simulink model "ClimaManModMgr"  (R2022b) (continued)
== system ClimaManModMgr == (continued)
  ClimaAutCtrldFlowPredForRowSecAtFlrAtStaby_ClimaAutCtrldFlowPredForRowSecAtFlrAtStaby/1 -> ClimaManModMgr_Runnable_sys/14(ClimaAutCtrldFlowPredForRowSecAtFlrAtStaby_ClimaAutCtrldFlowPredForRowSecAtFlrAtStaby_read)
  ClimaAutCtrldFlowPredForRowSecAtFlrNoLim_ClimaAutCtrldFlowPredForRowSecAtFlrNoLim/1 -> ClimaManModMgr_Runnable_sys/15(ClimaAutCtrldFlowPredForRowSecAtFlrNoLim_ClimaAutCtrldFlowPredForRowSecAtFlrNoLim_read)
  ClimaAutCtrldFlowPredForRowSecAtVentAtStaby_ClimaAutCtrldFlowPredForRowSecAtVentAtStaby/1 -> ClimaManModMgr_Runnable_sys/16(ClimaAutCtrldFlowPredForRowSecAtVentAtStaby_ClimaAutCtrldFlowPredForRowSecAtVentAtStaby_read)
  ClimaAutCtrldFlowPredForRowSecAtVentNoLim_ClimaAutCtrldFlowPredForRowSecAtVentNoLim/1 -> ClimaManModMgr_Runnable_sys/17(ClimaAutCtrldFlowPredForRowSecAtVentNoLim_ClimaAutCtrldFlowPredForRowSecAtVentNoLim_read)
  ClimaAutCtrldFlowReqForDefrst_ClimaAutCtrldFlowReqForDefrst/1 -> ClimaManModMgr_Runnable_sys/18(ClimaAutCtrldFlowReqForDefrst_ClimaAutCtrldFlowReqForDefrst_read)
  ClimaAutCtrldFlowReqForDefrst_ClimaAutCtrldFlowReqForDefrstLe/1 -> ClimaManModMgr_Runnable_sys/19(ClimaAutCtrldFlowReqForDefrst_ClimaAutCtrldFlowReqForDefrstLe_read)
  ClimaAutCtrldFlowReqForDefrst_ClimaAutCtrldFlowReqForDefrstRi/1 -> ClimaManModMgr_Runnable_sys/20(ClimaAutCtrldFlowReqForDefrst_ClimaAutCtrldFlowReqForDefrstRi_read)
  ClimaAutCtrldFlowReqForRowFirstAtFlr_ClimaAutCtrldFlowReqForRowFirstAtFlr/1 -> ClimaManModMgr_Runnable_sys/21(ClimaAutCtrldFlowReqForRowFirstAtFlr_ClimaAutCtrldFlowReqForRowFirstAtFlr_read)
  ClimaAutCtrldFlowReqForRowFirstAtFlr_ClimaAutCtrldFlowReqForRowFirstAtFlrRi/1 -> ClimaManModMgr_Runnable_sys/22(ClimaAutCtrldFlowReqForRowFirstAtFlr_ClimaAutCtrldFlowReqForRowFirstAtFlrRi_read)
  ClimaAutCtrldFlowReqForRowSecAtFlr_ClimaAutCtrldFlowReqForRowSecAtFlr/1 -> ClimaManModMgr_Runnable_sys/23(ClimaAutCtrldFlowReqForRowSecAtFlr_ClimaAutCtrldFlowReqForRowSecAtFlr_read)
  ClimaAutCtrldFlowReqForRowSecAtFlr_ClimaAutCtrldFlowReqForRowSecAtFlrLe/1 -> ClimaManModMgr_Runnable_sys/24(ClimaAutCtrldFlowReqForRowSecAtFlr_ClimaAutCtrldFlowReqForRowSecAtFlrLe_read)
  ClimaAutCtrldFlowReqForRowSecAtFlr_ClimaAutCtrldFlowReqForRowSecAtFlrRi/1 -> ClimaManModMgr_Runnable_sys/25(ClimaAutCtrldFlowReqForRowSecAtFlr_ClimaAutCtrldFlowReqForRowSecAtFlrRi_read)
  ClimaAutCtrldFlowReqForRowSecAtVent_ClimaAutCtrldFlowReqForRowSecAtVent/1 -> ClimaManModMgr_Runnable_sys/26(ClimaAutCtrldFlowReqForRowSecAtVent_ClimaAutCtrldFlowReqForRowSecAtVent_read)
  ClimaAutCtrldFlowReqForRowSecAtVent_ClimaAutCtrldFlowReqForRowSecAtVentLe/1 -> ClimaManModMgr_Runnable_sys/27(ClimaAutCtrldFlowReqForRowSecAtVent_ClimaAutCtrldFlowReqForRowSecAtVentLe_read)
  ClimaAutCtrldFlowReqForRowSecAtVent_ClimaAutCtrldFlowReqForRowSecAtVentRi/1 -> ClimaManModMgr_Runnable_sys/28(ClimaAutCtrldFlowReqForRowSecAtVent_ClimaAutCtrldFlowReqForRowSecAtVentRi_read)
  ClimaCfgForCarTyp1_ClimaCfgForCarTyp1/1 -> ClimaManModMgr_Runnable_sys/29(ClimaCfgForCarTyp1_ClimaCfgForCarTyp1_read)
  ClimaCfgForClimaCtrlMan1_ClimaCfgForClimaCtrlMan1/1 -> ClimaManModMgr_Runnable_sys/30(ClimaCfgForClimaCtrlMan1_ClimaCfgForClimaCtrlMan1_read)
  ClimaCfgForExtVentnClimaCtrl_ClimaCfgForExtVentnClimaCtrl/1 -> ClimaManModMgr_Runnable_sys/31(ClimaCfgForExtVentnClimaCtrl_ClimaCfgForExtVentnClimaCtrl_read)
  ClimaCfgForFanPotiCtrld1_ClimaCfgForFanPotiCtrld1/1 -> ClimaManModMgr_Runnable_sys/32(ClimaCfgForFanPotiCtrld1_ClimaCfgForFanPotiCtrld1_read)
  ClimaCfgForHeatrTyp1_ClimaCfgForHeatrTyp1/1 -> ClimaManModMgr_Runnable_sys/33(ClimaCfgForHeatrTyp1_ClimaCfgForHeatrTyp1_read)
  ClimaCfgForNrOfFanLvlSetg1_ClimaCfgForNrOfFanLvlSetg1/1 -> ClimaManModMgr_Runnable_sys/34(ClimaCfgForNrOfFanLvlSetg1_ClimaCfgForNrOfFanLvlSetg1_read)
  ClimaCfgForNrOfTSetg1_ClimaCfgForNrOfTSetg1/1 -> ClimaManModMgr_Runnable_sys/35(ClimaCfgForNrOfTSetg1_ClimaCfgForNrOfTSetg1_read)
  ClimaCfgForVehElec1_ClimaCfgForVehElec1/1 -> ClimaManModMgr_Runnable_sys/36(ClimaCfgForVehElec1_ClimaCfgForVehElec1_read)
  ClimaCfgForVehHev1_ClimaCfgForVehHev1/1 -> ClimaManModMgr_Runnable_sys/37(ClimaCfgForVehHev1_ClimaCfgForVehHev1_read)
  ClimaCfgForVehHyb1_ClimaCfgForVehHyb1/1 -> ClimaManModMgr_Runnable_sys/38(ClimaCfgForVehHyb1_ClimaCfgForVehHyb1_read)
  ClimaCtrlActvNgcc_ClimaCtrlActv/1 -> ClimaManModMgr_Runnable_sys/39(ClimaCtrlActvNgcc_ClimaCtrlActv_read)
  ClimaCtrlForEngStbActv_ClimaCtrlForEngStbActv/1 -> ClimaManModMgr_Runnable_sys/40(ClimaCtrlForEngStbActv_ClimaCtrlForEngStbActv_read)
  ClimaStStrtUp_ClimaStStrtUp/1 -> ClimaManModMgr_Runnable_sys/41(ClimaStStrtUp_ClimaStStrtUp_read)
  CmptmtAirTEstimdAtRowFirst_CmptmtAirTEstimdAtRowFirst/1 -> ClimaManModMgr_Runnable_sys/42(CmptmtAirTEstimdAtRowFirst_CmptmtAirTEstimdAtRowFirst_read)
  CmptmtAirTEstimdAtRowSec_CmptmtAirTEstimdAtRowSec/1 -> ClimaManModMgr_Runnable_sys/43(CmptmtAirTEstimdAtRowSec_CmptmtAirTEstimdAtRowSec_read)
  CnvincModActv_CnvincModActv/1 -> ClimaManModMgr_Runnable_sys/44(CnvincModActv_CnvincModActv_read)
  CooltTInHeatElm_CooltTInHeatElm/1 -> ClimaManModMgr_Runnable_sys/45(CooltTInHeatElm_CooltTInHeatElm_read)
  DrvrLeElecAirVentnSts_DrvrLeElecAirVentnSts/1 -> ClimaManModMgr_Runnable_sys/46(DrvrLeElecAirVentnSts_DrvrLeElecAirVentnSts_read)
  DrvrRiElecAirVentnSts_DrvrRiElecAirVentnSts/1 -> ClimaManModMgr_Runnable_sys/47(DrvrRiElecAirVentnSts_DrvrRiElecAirVentnSts_read)
  EcoModForClimaActv_EcoModForClimaActv/1 -> ClimaManModMgr_Runnable_sys/48(EcoModForClimaActv_EcoModForClimaActv_read)
  ExtVentFanVolStsFrmClima_ExtVentFanVolStsFrmClima/1 -> ClimaManModMgr_Runnable_sys/49(ExtVentFanVolStsFrmClima_ExtVentFanVolStsFrmClima_read)
  ExtVentStsFrmClima_ExtVentStsFrmClima/1 -> ClimaManModMgr_Runnable_sys/50(ExtVentStsFrmClima_ExtVentStsFrmClima_read)
  FlowRednAtPwrAllwdLo_FlowRednAtPwrAllwdLo/1 -> ClimaManModMgr_Runnable_sys/51(FlowRednAtPwrAllwdLo_FlowRednAtPwrAllwdLo_read)
  FlowRednAtPwrLvlLo_FlowRednAtPwrLvlLo/1 -> ClimaManModMgr_Runnable_sys/52(FlowRednAtPwrLvlLo_FlowRednAtPwrLvlLo_read)
  HmiCmptmtAirDistbnFrntForClima_HmiCmptmtAirDistbnFrntForClima/1 -> ClimaManModMgr_Runnable_sys/53(HmiCmptmtAirDistbnFrntForClima_HmiCmptmtAirDistbnFrntForClima_read)
  HmiCmptmtAirDistbnFrntForClima_HmiCmptmtAirDistbnFrntRiForClima/1 -> ClimaManModMgr_Runnable_sys/54(HmiCmptmtAirDistbnFrntForClima_HmiCmptmtAirDistbnFrntRiForClima_read)
  HmiCmptmtAirDistbnFrntForClima_HmiCmptmtAirDistbnReForClima/1 -> ClimaManModMgr_Runnable_sys/55(HmiCmptmtAirDistbnFrntForClima_HmiCmptmtAirDistbnReForClima_read)
  HmiCmptmtAirDistbnFrntForClima_HmiCmptmtAirDistbnReRiForClima/1 -> ClimaManModMgr_Runnable_sys/56(HmiCmptmtAirDistbnFrntForClima_HmiCmptmtAirDistbnReRiForClima_read)
  HmiDefrstMaxReqForClima_HmiDefrstMaxReqForClima/1 -> ClimaManModMgr_Runnable_sys/57(HmiDefrstMaxReqForClima_HmiDefrstMaxReqForClima_read)
  HmiHvacFanLvlFrntForClima_HmiHvacFanLvlFrntForClima/1 -> ClimaManModMgr_Runnable_sys/58(HmiHvacFanLvlFrntForClima_HmiHvacFanLvlFrntForClima_read)
  HmiHvacFanLvlFrntForClima_HmiHvacFanLvlFrntRiForClima/1 -> ClimaManModMgr_Runnable_sys/59(HmiHvacFanLvlFrntForClima_HmiHvacFanLvlFrntRiForClima_read)
  HmiHvacFanLvlReForClima_HmiHvacFanLvlReForClima/1 -> ClimaManModMgr_Runnable_sys/60(HmiHvacFanLvlReForClima_HmiHvacFanLvlReForClima_read)
  HmiHvacFanLvlReForClima_HmiHvacFanLvlReRiForClima/1 -> ClimaManModMgr_Runnable_sys/61(HmiHvacFanLvlReForClima_HmiHvacFanLvlReRiForClima_read)
  HmiMaxACReqForClima_HmiMaxACReqForClima/1 -> ClimaManModMgr_Runnable_sys/62(HmiMaxACReqForClima_HmiMaxACReqForClima_read)
  HvacAirMFlowReqMaxAvl_HvacAirMFlowReqMaxAvl/1 -> ClimaManModMgr_Runnable_sys/63(HvacAirMFlowReqMaxAvl_HvacAirMFlowReqMaxAvl_read)
  HvacAirTForRowFirstAtFlrLe_HvacAirTForRowFirstAtFlrLe_HvacAirTForRowFirstAtFlrLe/1 -> ClimaManModMgr_Runnable_sys/64(HvacAirTForRowFirstAtFlrLe_HvacAirTForRowFirstAtFlrLe_HvacAirTForRowFirstAtFlrLe_read)
  HvacAirTForRowFirstAtFlrLeRaw_HvacAirTForRowFirstAtFlrLeRaw/1 -> ClimaManModMgr_Runnable_sys/65(HvacAirTForRowFirstAtFlrLeRaw_HvacAirTForRowFirstAtFlrLeRaw_read)
  PassLeElecAirVentnSts_PassLeElecAirVentnSts/1 -> ClimaManModMgr_Runnable_sys/66(PassLeElecAirVentnSts_PassLeElecAirVentnSts_read)
  PassRiElecAirVentnSts_PassRiElecAirVentnSts/1 -> ClimaManModMgr_Runnable_sys/67(PassRiElecAirVentnSts_PassRiElecAirVentnSts_read)
  PreClngSts_PreClngSts/1 -> ClimaManModMgr_Runnable_sys/68(PreClngSts_PreClngSts_read)
  PrkgClimaActv_PrkgClimaActv/1 -> ClimaManModMgr_Runnable_sys/69(PrkgClimaActv_PrkgClimaActv_read)
  ReLeElecAirVentnSts_ReLeElecAirVentnSts/1 -> ClimaManModMgr_Runnable_sys/70(ReLeElecAirVentnSts_ReLeElecAirVentnSts_read)
  ReRiElecAirVentnSts_ReRiElecAirVentnSts/1 -> ClimaManModMgr_Runnable_sys/71(ReRiElecAirVentnSts_ReRiElecAirVentnSts_read)
  TModCtrlAtRowFirst_TModCtrlAtRowFirst/1 -> ClimaManModMgr_Runnable_sys/72(TModCtrlAtRowFirst_TModCtrlAtRowFirst_read)
  TModCtrlAtRowFirst_TModCtrlAtRowFirstLe/1 -> ClimaManModMgr_Runnable_sys/73(TModCtrlAtRowFirst_TModCtrlAtRowFirstLe_read)
  TModCtrlAtRowFirst_TModCtrlAtRowFirstRi/1 -> ClimaManModMgr_Runnable_sys/74(TModCtrlAtRowFirst_TModCtrlAtRowFirstRi_read)
  VehModMngtGlbSafe1_Ngcc_VehModMngtGlbSafe1/1 -> ClimaManModMgr_Runnable_sys/75(VehModMngtGlbSafe1_Ngcc_VehModMngtGlbSafe1_read)
  VentAndFlrModelForRowFirstLeForModelDial_VentAndFlrModelForRowFirstLeForModelDial/1 -> ClimaManModMgr_Runnable_sys/76(VentAndFlrModelForRowFirstLeForModelDial_VentAndFlrModelForRowFirstLeForModelDial_read)
  VentAndFlrModelForRowFirstRiForModelDial_VentAndFlrModelForRowFirstRiForModelDial/1 -> ClimaManModMgr_Runnable_sys/77(VentAndFlrModelForRowFirstRiForModelDial_VentAndFlrModelForRowFirstRiForModelDial_read)
  VentAndFlrModelForRowSecLeForModelDial_VentAndFlrModelForRowSecLeForModelDial/1 -> ClimaManModMgr_Runnable_sys/78(VentAndFlrModelForRowSecLeForModelDial_VentAndFlrModelForRowSecLeForModelDial_read)
  VentAndFlrModelForRowSecRiForModelDial_VentAndFlrModelForRowSecRiForModelDial/1 -> ClimaManModMgr_Runnable_sys/79(VentAndFlrModelForRowSecRiForModelDial_VentAndFlrModelForRowSecRiForModelDial_read)
  AirTFromHeatgEstimdForCCM_AirTFromHeatgEstimd/1 -> ClimaManModMgr_Runnable_sys/80(AirTFromHeatgEstimdForCCM_AirTFromHeatgEstimd_read)
  AutDemstOperSts_AutDemstOperSts/1 -> ClimaManModMgr_Runnable_sys/81(AutDemstOperSts_AutDemstOperSts_read)
  CdsnProblty_CdsnProblty/1 -> ClimaManModMgr_Runnable_sys/82(CdsnProblty_CdsnProblty_read)
  ClimaAutCtrldFlowReqForRowFirstAtFlr_ClimaAutCtrldFlowReqForRowFirstAtFlrLe/1 -> ClimaManModMgr_Runnable_sys/83(ClimaAutCtrldFlowReqForRowFirstAtFlr_ClimaAutCtrldFlowReqForRowFirstAtFlrLe_read)
  ClimaAutCtrldFlowReqForRowFirstAtVent_ClimaAutCtrldFlowReqForRowFirstAtVent/1 -> ClimaManModMgr_Runnable_sys/84(ClimaAutCtrldFlowReqForRowFirstAtVent_ClimaAutCtrldFlowReqForRowFirstAtVent_read)
  ClimaAutCtrldFlowReqForRowFirstAtVent_ClimaAutCtrldFlowReqForRowFirstAtVentLe/1 -> ClimaManModMgr_Runnable_sys/85(ClimaAutCtrldFlowReqForRowFirstAtVent_ClimaAutCtrldFlowReqForRowFirstAtVentLe_read)
  ClimaAutCtrldFlowReqForRowFirstAtVent_ClimaAutCtrldFlowReqForRowFirstAtVentLeMid/1 -> ClimaManModMgr_Runnable_sys/86(ClimaAutCtrldFlowReqForRowFirstAtVent_ClimaAutCtrldFlowReqForRowFirstAtVentLeMid_read)
  ClimaAutCtrldFlowReqForRowFirstAtVent_ClimaAutCtrldFlowReqForRowFirstAtVentLeSide/1 -> ClimaManModMgr_Runnable_sys/87(ClimaAutCtrldFlowReqForRowFirstAtVent_ClimaAutCtrldFlowReqForRowFirstAtVentLeSide_read)
  ClimaAutCtrldFlowReqForRowFirstAtVent_ClimaAutCtrldFlowReqForRowFirstAtVentRi/1 -> ClimaManModMgr_Runnable_sys/88(ClimaAutCtrldFlowReqForRowFirstAtVent_ClimaAutCtrldFlowReqForRowFirstAtVentRi_read)
  ClimaAutCtrldFlowReqForRowFirstAtVent_ClimaAutCtrldFlowReqForRowFirstAtVentRiMid/1 -> ClimaManModMgr_Runnable_sys/89(ClimaAutCtrldFlowReqForRowFirstAtVent_ClimaAutCtrldFlowReqForRowFirstAtVentRiMid_read)
  ClimaAutCtrldFlowReqForRowFirstAtVent_ClimaAutCtrldFlowReqForRowFirstAtVentRiSide/1 -> ClimaManModMgr_Runnable_sys/90(ClimaAutCtrldFlowReqForRowFirstAtVent_ClimaAutCtrldFlowReqForRowFirstAtVentRiSide_read)
  ClimaCfgForActrModVrnt1_ClimaCfgForActrInd1/1 -> ClimaManModMgr_Runnable_sys/91(ClimaCfgForActrModVrnt1_ClimaCfgForActrInd1_read)
  ClimaCfgForActrModVrnt1_ClimaCfgForActrModVrnt1/1 -> ClimaManModMgr_Runnable_sys/92(ClimaCfgForActrModVrnt1_ClimaCfgForActrModVrnt1_read)
  ClimaCfgForActrModVrnt1_ClimaCfgForActrModVrntCen/1 -> ClimaManModMgr_Runnable_sys/93(ClimaCfgForActrModVrnt1_ClimaCfgForActrModVrntCen_read)
  ClimaCfgForStatrtDevice_ClimaCfgForStartDevice/1 -> ClimaManModMgr_Runnable_sys/94(ClimaCfgForStatrtDevice_ClimaCfgForStartDevice_read)
  FacOfSecVent_FacOfSecVent/1 -> ClimaManModMgr_Runnable_sys/95(FacOfSecVent_FacOfSecVent_read)
  FacOfVentLeFlow_FacOfVentLeFlow/1 -> ClimaManModMgr_Runnable_sys/96(FacOfVentLeFlow_FacOfVentLeFlow_read)
  FacOfVentRiFlow_FacOfVentRiFlow/1 -> ClimaManModMgr_Runnable_sys/97(FacOfVentRiFlow_FacOfVentRiFlow_read)
  FacOfVentSideLeFlow_FacOfVentSideLeFlow/1 -> ClimaManModMgr_Runnable_sys/98(FacOfVentSideLeFlow_FacOfVentSideLeFlow_read)
  FacOfVentSideRiFlow_FacOfVentSideRiFlow/1 -> ClimaManModMgr_Runnable_sys/99(FacOfVentSideRiFlow_FacOfVentSideRiFlow_read)
  FlowRednAtPwrLvlLo1_FlowRednAtPwrLvlLo1/1 -> ClimaManModMgr_Runnable_sys/100(FlowRednAtPwrLvlLo1_FlowRednAtPwrLvlLo1_read)
  HmiCmptmtTSpForClima_HmiCmptmtTSpForClima/1 -> ClimaManModMgr_Runnable_sys/101(HmiCmptmtTSpForClima_HmiCmptmtTSpForClima_read)
  CmptmtTFrntRaw_CmptmtTFrntRaw/1 -> ClimaManModMgr_Runnable_sys/102(CmptmtTFrntRaw_CmptmtTFrntRaw_read)
  ActrFrntReqForRowFirstLeModDistbn_ActrFrntReqForRowFirstLeModDistbn/1 -> ClimaManModMgr_Runnable_sys/103(ActrFrntReqForRowFirstLeModDistbn_ActrFrntReqForRowFirstLeModDistbn_read)
  ActrFrntReqForRowFirstModDistbn_ActrFrntReqForRowFirstModDistbn/1 -> ClimaManModMgr_Runnable_sys/104(ActrFrntReqForRowFirstModDistbn_ActrFrntReqForRowFirstModDistbn_read)
  ActrPosnActForModDistbnRe_ActrPosnActForModDistbnRe/1 -> ClimaManModMgr_Runnable_sys/105(ActrPosnActForModDistbnRe_ActrPosnActForModDistbnRe_read)
  ActrPosnFrntActForRowFirstModDistbn_ActrPosnFrntActForRowFirstModDistbn/1 -> ClimaManModMgr_Runnable_sys/106(ActrPosnFrntActForRowFirstModDistbn_ActrPosnFrntActForRowFirstModDistbn_read)
  ActrPosnFrntActForRowFirstModDistbnLe_ActrPosnFrntActForRowFirstModDistbnLe/1 -> ClimaManModMgr_Runnable_sys/107(ActrPosnFrntActForRowFirstModDistbnLe_ActrPosnFrntActForRowFirstModDistbnLe_read)
  ActvnTiForClimaCtrlRe_ActvnTiForClimaCtrlRe/1 -> ClimaManModMgr_Runnable_sys/108(ActvnTiForClimaCtrlRe_ActvnTiForClimaCtrlRe_read)
  AirCdnAvl_AirCdnAvl/1 -> ClimaManModMgr_Runnable_sys/109(AirCdnAvl_AirCdnAvl_read)
  AirPtcEnadNgcc_AirPtcEnadNgcc/1 -> ClimaManModMgr_Runnable_sys/110(AirPtcEnadNgcc_AirPtcEnadNgcc_read)
  AirPtcModeReqNgcc_AirPtcModeReqNgcc/1 -> ClimaManModMgr_Runnable_sys/111(AirPtcModeReqNgcc_AirPtcModeReqNgcc_read)
  AirTAftHvacDesFirstLe_AirTAftHvacDesFirstLe/1 -> ClimaManModMgr_Runnable_sys/112(AirTAftHvacDesFirstLe_AirTAftHvacDesFirstLe_read)
  AirTAftHvacDesFirstRi_AirTAftHvacDesFirstRi/1 -> ClimaManModMgr_Runnable_sys/113(AirTAftHvacDesFirstRi_AirTAftHvacDesFirstRi_read)
  AirTAftHvacDesRe_AirTAftHvacDesRe/1 -> ClimaManModMgr_Runnable_sys/114(AirTAftHvacDesRe_AirTAftHvacDesRe_read)
  AirTAftHvacDesSecLe_AirTAftHvacDesSecLe/1 -> ClimaManModMgr_Runnable_sys/115(AirTAftHvacDesSecLe_AirTAftHvacDesSecLe_read)
  AirTFromHeatgEstimdFirstLeForCCM_AirTFromHeatgEstimdFirstLeForCCM/1 -> ClimaManModMgr_Runnable_sys/116(AirTFromHeatgEstimdFirstLeForCCM_AirTFromHeatgEstimdFirstLeForCCM_read)
  AirTFromHeatgEstimdFirstRiForCCM_AirTFromHeatgEstimdFirstRiForCCM/1 -> ClimaManModMgr_Runnable_sys/117(AirTFromHeatgEstimdFirstRiForCCM_AirTFromHeatgEstimdFirstRiForCCM_read)
  AirTFromHeatgEstimdReForCCM_AirTFromHeatgEstimdReForCCM/1 -> ClimaManModMgr_Runnable_sys/118(AirTFromHeatgEstimdReForCCM_AirTFromHeatgEstimdReForCCM_read)
  AirTFromHeatgEstimdSecLeForCCM_AirTFromHeatgEstimdSecLeForCCM/1 -> ClimaManModMgr_Runnable_sys/119(AirTFromHeatgEstimdSecLeForCCM_AirTFromHeatgEstimdSecLeForCCM_read)
  AutDemstWthFanReq_AutDemstWthFanReq/1 -> ClimaManModMgr_Runnable_sys/120(AutDemstWthFanReq_AutDemstWthFanReq_read)
  ClimaCfgForAutDemst_ClimaCfgForAutDemst/1 -> ClimaManModMgr_Runnable_sys/121(ClimaCfgForAutDemst_ClimaCfgForAutDemst_read)
  ClimaCfgForErev1_ClimaCfgForErev1/1 -> ClimaManModMgr_Runnable_sys/122(ClimaCfgForErev1_ClimaCfgForErev1_read)
  ClimaCfgForForHeatPmp_ClimaCfgForForHeatPmp/1 -> ClimaManModMgr_Runnable_sys/123(ClimaCfgForForHeatPmp_ClimaCfgForForHeatPmp_read)
  ClimaCfgForHvacRePrsnt1_ClimaCfgForHvacRePrsnt1/1 -> ClimaManModMgr_Runnable_sys/124(ClimaCfgForHvacRePrsnt1_ClimaCfgForHvacRePrsnt1_read)
  ClimaCfgForPTCInFrontHVAC_ClimaCfgForPTCInFrontHVAC/1 -> ClimaManModMgr_Runnable_sys/125(ClimaCfgForPTCInFrontHVAC_ClimaCfgForPTCInFrontHVAC_read)
  ClimaCfgForSnsrHumPrsnt1_ClimaCfgForSnsrHumPrsnt1/1 -> ClimaManModMgr_Runnable_sys/126(ClimaCfgForSnsrHumPrsnt1_ClimaCfgForSnsrHumPrsnt1_read)
  ClimaCfgHvacFrntForRowSecForActrTLe_ClimaCfgHvacFrntForRowSecForActrTLe/1 -> ClimaManModMgr_Runnable_sys/127(ClimaCfgHvacFrntForRowSecForActrTLe_ClimaCfgHvacFrntForRowSecForActrTLe_read)
  ClimaCfgHvacFrntForRowSecForActrTRi_ClimaCfgHvacFrntForRowSecForActrTRi/1 -> ClimaManModMgr_Runnable_sys/128(ClimaCfgHvacFrntForRowSecForActrTRi_ClimaCfgHvacFrntForRowSecForActrTRi_read)
  ClimaCfgReqVersionReplace_ClimaCfgReqVersionReplace/1 -> ClimaManModMgr_Runnable_sys/129(ClimaCfgReqVersionReplace_ClimaCfgReqVersionReplace_read)
  ClimaStStrtUpRe_ClimaStStrtUpRe/1 -> ClimaManModMgr_Runnable_sys/130(ClimaStStrtUpRe_ClimaStStrtUpRe_read)
  CmptmtAirTEstimdExtd_Ngcc_CmptmtAirTEstimdExtd/1 -> ClimaManModMgr_Runnable_sys/131(CmptmtAirTEstimdExtd_Ngcc_CmptmtAirTEstimdExtd_read)
  CmptmtTRearRaw_CmptmtTRearRaw/1 -> ClimaManModMgr_Runnable_sys/132(CmptmtTRearRaw_CmptmtTRearRaw_read)
  CoupldCtrlErrAtRowFirstAvg_CoupldCtrlErrAtRowFirstAvg/1 -> ClimaManModMgr_Runnable_sys/133(CoupldCtrlErrAtRowFirstAvg_CoupldCtrlErrAtRowFirstAvg_read)
  CoupldCtrlErrAtRowFirstLeNgcc_CoupldCtrlErrAtRowFirstLe/1 -> ClimaManModMgr_Runnable_sys/134(CoupldCtrlErrAtRowFirstLeNgcc_CoupldCtrlErrAtRowFirstLe_read)
  CoupldCtrlErrAtRowFirstRiNgcc_CoupldCtrlErrAtRowFirstRi/1 -> ClimaManModMgr_Runnable_sys/135(CoupldCtrlErrAtRowFirstRiNgcc_CoupldCtrlErrAtRowFirstRi_read)
  CoupldCtrlErrAtRowSecAvg_CoupldCtrlErrAtRowSecAvg/1 -> ClimaManModMgr_Runnable_sys/136(CoupldCtrlErrAtRowSecAvg_CoupldCtrlErrAtRowSecAvg_read)
  CoupldCtrlErrAtRowSecLeNgcc_CoupldCtrlErrAtRowSecLe/1 -> ClimaManModMgr_Runnable_sys/137(CoupldCtrlErrAtRowSecLeNgcc_CoupldCtrlErrAtRowSecLe_read)
  CoupldCtrlErrAtRowSecRiNgcc_CoupldCtrlErrAtRowSecRi/1 -> ClimaManModMgr_Runnable_sys/138(CoupldCtrlErrAtRowSecRiNgcc_CoupldCtrlErrAtRowSecRi_read)
  DCDCPwrAllwdForHvacFanRe_DCDCPwrAllwdForHvacFanRe/1 -> ClimaManModMgr_Runnable_sys/139(DCDCPwrAllwdForHvacFanRe_DCDCPwrAllwdForHvacFanRe_read)
  DropTopPosnStsNgcc_DropTopPosnStsNgcc/1 -> ClimaManModMgr_Runnable_sys/140(DropTopPosnStsNgcc_DropTopPosnStsNgcc_read)
  EvaprTFrntFildForClima_EvaprTFrntFildForClima/1 -> ClimaManModMgr_Runnable_sys/141(EvaprTFrntFildForClima_EvaprTFrntFildForClima_read)
  EvaprTReFildForClima_EvaprTReFildForClima/1 -> ClimaManModMgr_Runnable_sys/142(EvaprTReFildForClima_EvaprTReFildForClima_read)
  ExtVentToastFrmClima_Prkg_ExtVentToastFrmClima/1 -> ClimaManModMgr_Runnable_sys/143(ExtVentToastFrmClima_Prkg_ExtVentToastFrmClima_read)
  FlowRednForEngStby_FlowRednForEngStby/1 -> ClimaManModMgr_Runnable_sys/144(FlowRednForEngStby_FlowRednForEngStby_read)
  FogFlashPrevnActv_FogFlashPrevnActv/1 -> ClimaManModMgr_Runnable_sys/145(FogFlashPrevnActv_FogFlashPrevnActv_read)
  FrntHvacBlowerSts_FrntHvacBlowerSts/1 -> ClimaManModMgr_Runnable_sys/146(FrntHvacBlowerSts_FrntHvacBlowerSts_read)
  HmiClimaFrntAutReq_HmiClimaFrntAutReq/1 -> ClimaManModMgr_Runnable_sys/147(HmiClimaFrntAutReq_HmiClimaFrntAutReq_read)
  HvacAirMFlowEstimd_Ngcc_HvacAirMFlowEstimd/1 -> ClimaManModMgr_Runnable_sys/148(HvacAirMFlowEstimd_Ngcc_HvacAirMFlowEstimd_read)
  HvacAirMFlowEstimdForCtrlr_HvacAirMFlowEstimdForCtrlr/1 -> ClimaManModMgr_Runnable_sys/149(HvacAirMFlowEstimdForCtrlr_HvacAirMFlowEstimdForCtrlr_read)
  HvacAirMFlowEstimdForCtrlrRe_HvacAirMFlowEstimdForCtrlrRe/1 -> ClimaManModMgr_Runnable_sys/150(HvacAirMFlowEstimdForCtrlrRe_HvacAirMFlowEstimdForCtrlrRe_read)
  HvacAirMFlowMaxEstimd_HvacAirMFlowMaxEstimd/1 -> ClimaManModMgr_Runnable_sys/151(HvacAirMFlowMaxEstimd_HvacAirMFlowMaxEstimd_read)
  HvacAirMFlowMaxEstimdRe_HvacAirMFlowMaxEstimdRe/1 -> ClimaManModMgr_Runnable_sys/152(HvacAirMFlowMaxEstimdRe_HvacAirMFlowMaxEstimdRe_read)
  HvacAirMFlowReEstimd_Ngcc_HvacAirMFlowReEstimd/1 -> ClimaManModMgr_Runnable_sys/153(HvacAirMFlowReEstimd_Ngcc_HvacAirMFlowReEstimd_read)
  HvacAirTEstimdAtRowFirstLe_HvacAirTEstimdAtRowFirstLe/1 -> ClimaManModMgr_Runnable_sys/154(HvacAirTEstimdAtRowFirstLe_HvacAirTEstimdAtRowFirstLe_read)
  HvacAirTEstimdAtRowFirstRi_HvacAirTEstimdAtRowFirstRi/1 -> ClimaManModMgr_Runnable_sys/155(HvacAirTEstimdAtRowFirstRi_HvacAirTEstimdAtRowFirstRi_read)
  HvacAirTEstimdAtRowSecLe_HvacAirTEstimdAtRowSecLe/1 -> ClimaManModMgr_Runnable_sys/156(HvacAirTEstimdAtRowSecLe_HvacAirTEstimdAtRowSecLe_read)
  HvacAirTEstimdAtRowSecRi_HvacAirTEstimdAtRowSecRi/1 -> ClimaManModMgr_Runnable_sys/157(HvacAirTEstimdAtRowSecRi_HvacAirTEstimdAtRowSecRi_read)
  HvacAirTFildForRowFirstAtDefrstLe_HvacAirTFildForRowFirstAtDefrstLe/1 -> ClimaManModMgr_Runnable_sys/158(HvacAirTFildForRowFirstAtDefrstLe_HvacAirTFildForRowFirstAtDefrstLe_read)
  HvacAirTFildForRowFirstAtDefrstRi_HvacAirTFildForRowFirstAtDefrstRi/1 -> ClimaManModMgr_Runnable_sys/159(HvacAirTFildForRowFirstAtDefrstRi_HvacAirTFildForRowFirstAtDefrstRi_read)
  HvacAirTFildForRowFirstAtDefrstSideLe_HvacAirTFildForRowFirstAtDefrstSideLe/1 -> ClimaManModMgr_Runnable_sys/160(HvacAirTFildForRowFirstAtDefrstSideLe_HvacAirTFildForRowFirstAtDefrstSideLe_read)
  HvacAirTFildForRowFirstAtDefrstSideRi_HvacAirTFildForRowFirstAtDefrstSideRi/1 -> ClimaManModMgr_Runnable_sys/161(HvacAirTFildForRowFirstAtDefrstSideRi_HvacAirTFildForRowFirstAtDefrstSideRi_read)
  HvacAirTReqAtRowFirstLe_HvacAirTReqAtRowFirstLe/1 -> ClimaManModMgr_Runnable_sys/162(HvacAirTReqAtRowFirstLe_HvacAirTReqAtRowFirstLe_read)
  HvacAirTReqAtRowFirstLe_HvacAirTReqAtRowFirstLe_IsUpdated/1 -> ClimaManModMgr_Runnable_sys/163(HvacAirTReqAtRowFirstLe_HvacAirTReqAtRowFirstLe_IsUpdated_read)
  HvacAirTReqAtRowFirstRi_HvacAirTReqAtRowFirstRi/1 -> ClimaManModMgr_Runnable_sys/164(HvacAirTReqAtRowFirstRi_HvacAirTReqAtRowFirstRi_read)
  HvacAirTReqAtRowFirstRi_HvacAirTReqAtRowFirstRi_IsUpdated/1 -> ClimaManModMgr_Runnable_sys/165(HvacAirTReqAtRowFirstRi_HvacAirTReqAtRowFirstRi_IsUpdated_read)
  HvacAirTReqAtRowSecLe_HvacAirTReqAtRowSecLe/1 -> ClimaManModMgr_Runnable_sys/166(HvacAirTReqAtRowSecLe_HvacAirTReqAtRowSecLe_read)
  HvacAirTReqAtRowSecLe_HvacAirTReqAtRowSecLe_IsUpdated/1 -> ClimaManModMgr_Runnable_sys/167(HvacAirTReqAtRowSecLe_HvacAirTReqAtRowSecLe_IsUpdated_read)
  HvacAirTReqAtRowSecRi_HvacAirTReqAtRowSecRi/1 -> ClimaManModMgr_Runnable_sys/168(HvacAirTReqAtRowSecRi_HvacAirTReqAtRowSecRi_read)
  HvacAirTReqAtRowSecRi_HvacAirTReqAtRowSecRi_IsUpdated/1 -> ClimaManModMgr_Runnable_sys/169(HvacAirTReqAtRowSecRi_HvacAirTReqAtRowSecRi_IsUpdated_read)
  HvacCtrlReqForActrModRe_HvacCtrlReqForActrModRe/1 -> ClimaManModMgr_Runnable_sys/170(HvacCtrlReqForActrModRe_HvacCtrlReqForActrModRe_read)
  HvacCtrlrReqFrntForRowFirstForActrMod_HvacCtrlrReqFrntForRowFirstForActrMod/1 -> ClimaManModMgr_Runnable_sys/171(HvacCtrlrReqFrntForRowFirstForActrMod_HvacCtrlrReqFrntForRowFirstForActrMod_read)
  HvacCtrlrReqFrntForRowFirstLeForActrMod_HvacCtrlrReqFrntForRowFirstLeForActrMod/1 -> ClimaManModMgr_Runnable_sys/172(HvacCtrlrReqFrntForRowFirstLeForActrMod_HvacCtrlrReqFrntForRowFirstLeForActrMod_read)
  HvacFanLvlFbFrnt_HvacFanLvlFbFrnt/1 -> ClimaManModMgr_Runnable_sys/173(HvacFanLvlFbFrnt_HvacFanLvlFbFrnt_read)
  HvacTarFlowTot_HvacTarFlowTot/1 -> ClimaManModMgr_Runnable_sys/174(HvacTarFlowTot_HvacTarFlowTot_read)
  ManModeForRowThrdForModelDial_ManModeForRowThrdForModelDial/1 -> ClimaManModMgr_Runnable_sys/175(ManModeForRowThrdForModelDial_ManModeForRowThrdForModelDial_read)
  PrkgClimaOnReq_PrkgClimaOnReq/1 -> ClimaManModMgr_Runnable_sys/176(PrkgClimaOnReq_PrkgClimaOnReq_read)
  PrkgClimaOnReqRe_PrkgClimaOnReqRe/1 -> ClimaManModMgr_Runnable_sys/177(PrkgClimaOnReqRe_PrkgClimaOnReqRe_read)
  ReHvacBlowerSts_ReHvacBlowerSts/1 -> ClimaManModMgr_Runnable_sys/178(ReHvacBlowerSts_ReHvacBlowerSts_read)
  ResrvdSigForECM4_ResrvdSigForECM4/1 -> ClimaManModMgr_Runnable_sys/179(ResrvdSigForECM4_ResrvdSigForECM4_read)
  RuranACModeSetNgcc_RuranACModeSetNgcc/1 -> ClimaManModMgr_Runnable_sys/180(RuranACModeSetNgcc_RuranACModeSetNgcc_read)
  SportModeThmgtRequestNgcc_SportModeThmgtRequestNgcc/1 -> ClimaManModMgr_Runnable_sys/181(SportModeThmgtRequestNgcc_SportModeThmgtRequestNgcc_read)
  VehSunLoadAtRowFirstLeFild_VehSunLoadAtRowFirstLeFild/1 -> ClimaManModMgr_Runnable_sys/182(VehSunLoadAtRowFirstLeFild_VehSunLoadAtRowFirstLeFild_read)
  VehSunLoadAtRowFirstRiFild_VehSunLoadAtRowFirstRiFild/1 -> ClimaManModMgr_Runnable_sys/183(VehSunLoadAtRowFirstRiFild_VehSunLoadAtRowFirstRiFild_read)
  VehSunLoadAtRowSecLeFild_VehSunLoadAtRowSecLeFild/1 -> ClimaManModMgr_Runnable_sys/184(VehSunLoadAtRowSecLeFild_VehSunLoadAtRowSecLeFild_read)
  VehSunLoadAtRowSecRiFild_VehSunLoadAtRowSecRiFild/1 -> ClimaManModMgr_Runnable_sys/185(VehSunLoadAtRowSecRiFild_VehSunLoadAtRowSecRiFild_read)
  ClimaManModMgr_Runnable_sys/1(AirFlowLimDurgMidAmbtForNVHForHVACFrntFlag_AirFlowLimDurgMidAmbtForNVHForHVACFrntFlag_write) -> AirFlowLimDurgMidAmbtForNVHForHVACFrntFlag_AirFlowLimDurgMidAmbtForNVHForHVACFrntFlag/1
  ClimaManModMgr_Runnable_sys/2(AirFlowLimDurgMidAmbtForNVHForHVACReFlag_AirFlowLimDurgMidAmbtForNVHForHVACReFlag_write) -> AirFlowLimDurgMidAmbtForNVHForHVACReFlag_AirFlowLimDurgMidAmbtForNVHForHVACReFlag/1
  ClimaManModMgr_Runnable_sys/3(AirTFromHeatgEstimdLimd_AirTFromHeatgEstimdLimd_write) -> AirTFromHeatgEstimdLimd_AirTFromHeatgEstimdLimd/1
  ClimaManModMgr_Runnable_sys/4(AirTFromHeatgEstimdLimdRe_AirTFromHeatgEstimdLimdRe_write) -> AirTFromHeatgEstimdLimdRe_AirTFromHeatgEstimdLimdRe/1
  ClimaManModMgr_Runnable_sys/5(AntiHotFlowToFaceActvFrnt_AntiHotFlowToFaceActvFrnt_write) -> AntiHotFlowToFaceActvFrnt_AntiHotFlowToFaceActvFrnt/1
  ClimaManModMgr_Runnable_sys/6(AntiHotFlowToFaceActvRe_AntiHotFlowToFaceActvRe_write) -> AntiHotFlowToFaceActvRe_AntiHotFlowToFaceActvRe/1
  ClimaManModMgr_Runnable_sys/7(ClimaManModCmftPopUpReqNgcc_ClimaManModCmftPopUpReqNgcc_write) -> ClimaManModCmftPopUpReqNgcc_ClimaManModCmftPopUpReqNgcc/1
  ClimaManModMgr_Runnable_sys/8(CmptmtAirDistbnRspNgcc_CmptmtAirDistbnRspNgcc_write) -> CmptmtAirDistbnRspNgcc_CmptmtAirDistbnRspNgcc/1
  ClimaManModMgr_Runnable_sys/9(FlowLimActv_FlowLimActv_write) -> FlowLimActv_FlowLimActv/1
  ClimaManModMgr_Runnable_sys/10(FlowLimActvRe_FlowLimActvRe_write) -> FlowLimActvRe_FlowLimActvRe/1
  ClimaManModMgr_Runnable_sys/11(FrntClimaManModCmftStsNgcc_FrntClimaManModCmftStsNgcc_write) -> FrntClimaManModCmftStsNgcc_FrntClimaManModCmftStsNgcc/1
  ClimaManModMgr_Runnable_sys/12(LowrWtrTActvn_LowrWtrTActvn_write) -> LowrWtrTActvn_LowrWtrTActvn/1
  ClimaManModMgr_Runnable_sys/13(ReClimaManModCmftStsNgcc_ReClimaManModCmftStsNgcc_write) -> ReClimaManModCmftStsNgcc_ReClimaManModCmftStsNgcc/1
  ClimaManModMgr_Runnable_sys/14(RuranModeSetAirFlowActv_RuranModeSetAirFlowActv_write) -> RuranModeSetAirFlowActv_RuranModeSetAirFlowActv/1
  ClimaManModMgr_Runnable_sys/15(ActrPosnReqForModDistbnForClimaCtrlMan_ActrPosnReqForModDistbnForClimaCtrlMan_write) -> ActrPosnReqForModDistbnForClimaCtrlMan_ActrPosnReqForModDistbnForClimaCtrlMan/1
  ClimaManModMgr_Runnable_sys/16(ClimaFlowPredCorrdForManModForDefrstAtStaby_ClimaFlowPredCorrdForManModForDefrstAtStaby_write) -> ClimaFlowPredCorrdForManModForDefrstAtStaby_ClimaFlowPredCorrdForManModForDefrstAtStaby/1
  ClimaManModMgr_Runnable_sys/17(ClimaFlowPredCorrdForManModForDefrstNoLim_ClimaFlowPredCorrdForManModForDefrstNoLim_write) -> ClimaFlowPredCorrdForManModForDefrstNoLim_ClimaFlowPredCorrdForManModForDefrstNoLim/1
  ClimaManModMgr_Runnable_sys/18(ClimaFlowPredCorrdForManModForRowFirstAtFlrAtStaby_ClimaFlowPredCorrdForManModForRowFirstAtFlrAtStaby_write) -> ClimaFlowPredCorrdForManModForRowFirstAtFlrAtStaby_ClimaFlowPredCorrdForManModForRowFirstAtFlrAtStaby/1
  ClimaManModMgr_Runnable_sys/19(ClimaFlowPredCorrdForManModForRowFirstAtFlrNoLim_ClimaFlowPredCorrdForManModForRowFirstAtFlrNoLim_write) -> ClimaFlowPredCorrdForManModForRowFirstAtFlrNoLim_ClimaFlowPredCorrdForManModForRowFirstAtFlrNoLim/1
  ClimaManModMgr_Runnable_sys/20(ClimaFlowPredCorrdForManModForRowFirstAtVentAtStaby_ClimaFlowPredCorrdForManModForRowFirstAtVentAtStaby_write) -> ClimaFlowPredCorrdForManModForRowFirstAtVentAtStaby_ClimaFlowPredCorrdForManModForRowFirstAtVentAtStaby/1
  ClimaManModMgr_Runnable_sys/21(ClimaFlowPredCorrdForManModForRowFirstAtVentNoLim_ClimaFlowPredCorrdForManModForRowFirstAtVentNoLim_write) -> ClimaFlowPredCorrdForManModForRowFirstAtVentNoLim_ClimaFlowPredCorrdForManModForRowFirstAtVentNoLim/1
  ClimaManModMgr_Runnable_sys/22(ClimaFlowPredCorrdForManModForRowSecAtFlrAtStaby_ClimaFlowPredCorrdForManModForRowSecAtFlrAtStaby_write) -> ClimaFlowPredCorrdForManModForRowSecAtFlrAtStaby_ClimaFlowPredCorrdForManModForRowSecAtFlrAtStaby/1
  ClimaManModMgr_Runnable_sys/23(ClimaFlowPredCorrdForManModForRowSecAtFlrNoLim_ClimaFlowPredCorrdForManModForRowSecAtFlrNoLim_write) -> ClimaFlowPredCorrdForManModForRowSecAtFlrNoLim_ClimaFlowPredCorrdForManModForRowSecAtFlrNoLim/1
  ClimaManModMgr_Runnable_sys/24(ClimaFlowPredCorrdForManModForRowSecAtVentAtStaby_ClimaFlowPredCorrdForManModForRowSecAtVentAtStaby_write) -> ClimaFlowPredCorrdForManModForRowSecAtVentAtStaby_ClimaFlowPredCorrdForManModForRowSecAtVentAtStaby/1
  ClimaManModMgr_Runnable_sys/25(ClimaFlowPredCorrdForManModForRowSecAtVentNoLim_ClimaFlowPredCorrdForManModForRowSecAtVentNoLim_write) -> ClimaFlowPredCorrdForManModForRowSecAtVentNoLim_ClimaFlowPredCorrdForManModForRowSecAtVentNoLim/1
  ClimaManModMgr_Runnable_sys/26(ClimaFlowReqCorrdForManModForDefrst_ClimaFlowReqCorrdForManModForDefrst_write) -> ClimaFlowReqCorrdForManModForDefrst_ClimaFlowReqCorrdForManModForDefrst/1
  ClimaManModMgr_Runnable_sys/27(ClimaFlowReqCorrdForManModForDefrst_ClimaFlowReqCorrdForManModForDefrstLe_write) -> ClimaFlowReqCorrdForManModForDefrst_ClimaFlowReqCorrdForManModForDefrstLe/1
  ClimaManModMgr_Runnable_sys/28(ClimaFlowReqCorrdForManModForDefrst_ClimaFlowReqCorrdForManModForDefrstRi_write) -> ClimaFlowReqCorrdForManModForDefrst_ClimaFlowReqCorrdForManModForDefrstRi/1
  ClimaManModMgr_Runnable_sys/29(ClimaFlowReqCorrdForManModForRowFirstAtFlr_ClimaFlowReqCorrdForManModForRowFirstAtFlr_write) -> ClimaFlowReqCorrdForManModForRowFirstAtFlr_ClimaFlowReqCorrdForManModForRowFirstAtFlr/1
  ClimaManModMgr_Runnable_sys/30(ClimaFlowReqCorrdForManModForRowFirstAtFlr_ClimaFlowReqCorrdForManModForRowFirstAtFlrLe_write) -> ClimaFlowReqCorrdForManModForRowFirstAtFlr_ClimaFlowReqCorrdForManModForRowFirstAtFlrLe/1
  ClimaManModMgr_Runnable_sys/31(ClimaFlowReqCorrdForManModForRowFirstAtFlr_ClimaFlowReqCorrdForManModForRowFirstAtFlrRi_write) -> ClimaFlowReqCorrdForManModForRowFirstAtFlr_ClimaFlowReqCorrdForManModForRowFirstAtFlrRi/1
  ClimaManModMgr_Runnable_sys/32(ClimaFlowReqCorrdForManModForRowFirstAtVent_ClimaFlowReqCorrdForManModForRowFirstAtVent_write) -> ClimaFlowReqCorrdForManModForRowFirstAtVent_ClimaFlowReqCorrdForManModForRowFirstAtVent/1
  ClimaManModMgr_Runnable_sys/33(ClimaFlowReqCorrdForManModForRowFirstAtVent_ClimaFlowReqCorrdForManModForRowFirstAtVentLe_write) -> ClimaFlowReqCorrdForManModForRowFirstAtVent_ClimaFlowReqCorrdForManModForRowFirstAtVentLe/1
  ClimaManModMgr_Runnable_sys/34(ClimaFlowReqCorrdForManModForRowFirstAtVent_ClimaFlowReqCorrdForManModForRowFirstAtVentLeMid_write) -> ClimaFlowReqCorrdForManModForRowFirstAtVent_ClimaFlowReqCorrdForManModForRowFirstAtVentLeMid/1
  ClimaManModMgr_Runnable_sys/35(ClimaFlowReqCorrdForManModForRowFirstAtVent_ClimaFlowReqCorrdForManModForRowFirstAtVentLeSide_write) -> ClimaFlowReqCorrdForManModForRowFirstAtVent_ClimaFlowReqCorrdForManModForRowFirstAtVentLeSide/1
  ClimaManModMgr_Runnable_sys/36(ClimaFlowReqCorrdForManModForRowFirstAtVent_ClimaFlowReqCorrdForManModForRowFirstAtVentRi_write) -> ClimaFlowReqCorrdForManModForRowFirstAtVent_ClimaFlowReqCorrdForManModForRowFirstAtVentRi/1
  ClimaManModMgr_Runnable_sys/37(ClimaFlowReqCorrdForManModForRowFirstAtVent_ClimaFlowReqCorrdForManModForRowFirstAtVentRiMid_write) -> ClimaFlowReqCorrdForManModForRowFirstAtVent_ClimaFlowReqCorrdForManModForRowFirstAtVentRiMid/1
  ClimaManModMgr_Runnable_sys/38(ClimaFlowReqCorrdForManModForRowFirstAtVent_ClimaFlowReqCorrdForManModForRowFirstAtVentRiSide_write) -> ClimaFlowReqCorrdForManModForRowFirstAtVent_ClimaFlowReqCorrdForManModForRowFirstAtVentRiSide/1
  ClimaManModMgr_Runnable_sys/39(ClimaFlowReqCorrdForManModForRowSecAtFlr_ClimaFlowReqCorrdForManModForRowSecAtFlr_write) -> ClimaFlowReqCorrdForManModForRowSecAtFlr_ClimaFlowReqCorrdForManModForRowSecAtFlr/1
  ClimaManModMgr_Runnable_sys/40(ClimaFlowReqCorrdForManModForRowSecAtFlr_ClimaFlowReqCorrdForManModForRowSecAtFlrLe_write) -> ClimaFlowReqCorrdForManModForRowSecAtFlr_ClimaFlowReqCorrdForManModForRowSecAtFlrLe/1
  ClimaManModMgr_Runnable_sys/41(ClimaFlowReqCorrdForManModForRowSecAtFlr_ClimaFlowReqCorrdForManModForRowSecAtFlrRi_write) -> ClimaFlowReqCorrdForManModForRowSecAtFlr_ClimaFlowReqCorrdForManModForRowSecAtFlrRi/1
  ClimaManModMgr_Runnable_sys/42(ClimaFlowReqCorrdForManModForRowSecAtVent_ClimaFlowReqCorrdForManModForRowSecAtVent_write) -> ClimaFlowReqCorrdForManModForRowSecAtVent_ClimaFlowReqCorrdForManModForRowSecAtVent/1
  ClimaManModMgr_Runnable_sys/43(ClimaFlowReqCorrdForManModForRowSecAtVent_ClimaFlowReqCorrdForManModForRowSecAtVentLe_write) -> ClimaFlowReqCorrdForManModForRowSecAtVent_ClimaFlowReqCorrdForManModForRowSecAtVentLe/1
  ClimaManModMgr_Runnable_sys/44(ClimaFlowReqCorrdForManModForRowSecAtVent_ClimaFlowReqCorrdForManModForRowSecAtVentRi_write) -> ClimaFlowReqCorrdForManModForRowSecAtVent_ClimaFlowReqCorrdForManModForRowSecAtVentRi/1
  ClimaManModMgr_Runnable_sys/45(FanManSetgActv_FanManSetgActv_write) -> FanManSetgActv_FanManSetgActv/1
  ClimaManModMgr_Runnable_sys/46(HvacFanSpdReqFrntForClimaCtrlMan_HvacFanSpdReqFrntForClimaCtrlMan_write) -> HvacFanSpdReqFrntForClimaCtrlMan_HvacFanSpdReqFrntForClimaCtrlMan/1
  ClimaManModMgr_Runnable_sys/47(ManModeForRowFirstLeForModelDial_ManModeForRowFirstLeForModelDial_write) -> ManModeForRowFirstLeForModelDial_ManModeForRowFirstLeForModelDial/1
  ClimaManModMgr_Runnable_sys/48(ManModeForRowFirstRiForModelDial_ManModeForRowFirstRiForModelDial_write) -> ManModeForRowFirstRiForModelDial_ManModeForRowFirstRiForModelDial/1
  ClimaManModMgr_Runnable_sys/49(ManModeForRowSecLeForModelDial_ManModeForRowSecLeForModelDial_write) -> ManModeForRowSecLeForModelDial_ManModeForRowSecLeForModelDial/1
  ClimaManModMgr_Runnable_sys/50(ManModeForRowSecRiForModelDial_ManModeForRowSecRiForModelDial_write) -> ManModeForRowSecRiForModelDial_ManModeForRowSecRiForModelDial/1
  ClimaManModMgr_Runnable/1 -> ClimaManModMgr_Runnable_sys/Trigger
